simulink library "Garbage"  (R2023a)
settings: Solver=ode45  StartTime=0.0  StopTime=10.0  RelTol=1e-3  EnableMultiTasking=on
undefined symbols (not defined in the file or in its folder): c, ending_column_index, ending_row_index, framebuffer_16x1, framebuffer_2x2, framebuffer_4x4, framebuffer_8x8, HEIGHT, hot, POS_X, POS_Y, starting_column_index, starting_row_index, WIDTH
libraries: Memory_Blocks_Lib x459, simulink x235, simulink_extras x132, dspmtrx3 x116, Bit_Multiplexers_Lib x67, Bit_Demultiplexers_Lib x49, Byte_Multiplexers_Lib x40, Miscellaneous x38, Computer_Library x17, Decoders_Lib x14, commutil2 x12, Arithmetic_Operations_Lib x8
(part 12 of 26)

== system Garbage/NOT_USED_Counter_4bit_Hours ==
  note: J3 = ~(20+).~Rst.Q0.~Q3.Q2.Q1
      | K3 = (20+) + Rst + Q0 + ~Q3 + Q2 + Q1
      |
      | J2 = ~(20+).~Rst.Q0.~Q3.~Q2.Q1
      | K2 = ~(20+).~Rst.Q0.~Q3.Q2.Q1 + Rst + (20+) + Q3
      |
      | J1 = ~Rst.Q0.~Q3.(~(20+) + (20+).~Q2)
      | K1 = ((20+) + Rst + Q0 + Q3).(~(20+) + Rst + Q0 + Q3 + Q2)
      |
      | J0 = ~(20+).~Rst.~Q0.(~Q3 + Q3.~Q2.~Q1) + (20+).~Rst.~Q3.~Q2
      | K0 = 1
  note: Present state
      |
      | Next state
      |
      | Hours < 20
      | Hours >= 20
      | Hours < 20
      | Hours >= 20
      |
      | Rst = 0
      | Rst = 1
      | Rst = 0
      | Rst = 1
      | Rst = 0
      | Rst = 1
      | Rst = 0
      | Rst = 1
      |
      | Q3
      | Q2
      | Q1
      | Q0
      | J3
      | K3
      | J2
      | K2
      | J1
      | K1
      | J0
      | K0
      | J3
      | K3
      | J2
      | K2
      | J1
      | K1
      | J0
      | K0
      | J3
      | K3
      | J2
      | K2
      | J1
      | K1
      | J0
      | K0
      | J3
      | K3
      | J2
      | K2
      | J1
      | K1
      | J0
      | K0
      | Q3
      | Q2
      | Q1
      | Q0
      | Q3
      | Q2
      | Q1
      | Q0
      | Q3
      | Q2
      | Q1
      | Q0
      | Q3
      | Q2
      | Q1
      | Q0
      |
      | 0
      | 0
      | 0
      | 0
      | 0
      | X
      | 0
      | X
      | 0
      | X
      | 1
      | X
      | 0
      | X
      | 0
      | X
      | 0
      | X
      | 0
      | X
      | 0
      | X
      | 0
      | X
      | 0
      | X
      | 1
      | X
      | 0
      | X
      | 0
      | X
      | 0
      | X
      | 0
      | X
      | 0
      | 0
      | 0
      | 1
      | 0
      | 0
      | 0
      | 0
      | 0
      | 0
      | 0
      | 1
      | 0
      | 0
      | 0
      | 0
      |
      | 0
      | 0
      | 0
      | 1
      | 0
      | X
      | 0
      | X
      | 1
      | X
      | X
      | 1
      | 0
      | X
      | 0
      | X
      | 0
      | X
      | X
      | 1
      | 0
      | X
      | 0
      | X
      | 1
      | X
      | X
      | 1
      | 0
      | X
      | 0
      | X
      | 0
      | X
      | X
      | 1
      | 0
      | 0
      | 1
      | 0
      | 0
      | 0
      | 0
      | 0
      | 0
      | 0
      | 1
      | 0
      | 0
      | 0
      | 0
      | 0
      |
      | 0
      | 0
      | 1
      | 0
      | 0
      | X
      | 0
      | X
      | X
      | 0
      | 1
      | X
      | 0
      | X
      | 0
      | X
      | X
      | 1
      | 0
      | X
      | 0
      | X
      | 0
      | X
      | X
      | 0
      | 1
      | X
      | 0
      | X
      | 0
      | X
      | X
      | 1
      | 0
      | X
      | 0
      | 0
      | 1
      | 1
      | 0
      | 0
      | 0
      | 0
      | 0
      | 0
      | 1
      | 1
      | 0
      | 0
      | 0
      | 0
      |
      | 0
      | 0
      | 1
      | 1
      | 0
      | X
      | 1
      | X
      | X
      | 1
      | X
      | 1
      | 0
      | X
      | 0
      | X
      | X
      | 1
      | X
      | 1
      | 0
      | X
      | 0
      | X
      | X
      | 1
      | X
      | 1
      | 0
      | X
      | 0
      | X
      | X
      | 1
      | X
      | 1
      | 0
      | 1
      | 0
      | 0
      | 0
      | 0
      | 0
      | 0
      | 0
      | 0
      | 0
      | 0
      | 0
      | 0
      | 0
      | 0
      |
      | 0
      | 1
      | 0
      | 0
      | 0
      | X
      | X
      | 0
      | 0
      | X
      | 1
      | X
      | 0
      | X
      | X
      | 1
      | 0
      | X
      | 0
      | X
      | 0
      | X
      | X
      | 1
      | 0
      | X
      | 0
      | X
      | 0
      | X
      | X
      | 1
      | 0
      | X
      | 0
      | X
      | 0
      | 1
      | 0
      | 1
      | 0
      | 0
      | 0
      | 0
      | 0
      | 0
      | 0
      | 0
      | 0
      | 0
      | 0
      | 0
      |
      | 0
      | 1
      | 0
      | 1
      | 0
      | X
      | X
      | 0
      | 1
      | X
      | X
      | 1
      | 0
      | X
      | X
      | 1
      | 0
      | X
      | X
      | 1
      | 0
      | X
      | X
      | 1
      | 0
      | X
      | X
      | 1
      | 0
      | X
      | X
      | 1
      | 0
      | X
      | X
      | 1
      | 0
      | 1
      | 1
      | 0
      | 0
      | 0
      | 0
      | 0
      | 0
      | 0
      | 0
      | 0
      | 0
      | 0
      | 0
      | 0
      |
      | 0
      | 1
      | 1
      | 0
      | 0
      | X
      | X
      | 0
      | X
      | 0
      | 1
      | X
      | 0
      | X
      | X
      | 1
      | X
      | 1
      | 0
      | X
      | 0
      | X
      | X
      | 1
      | X
      | 1
      | 0
      | X
      | 0
      | X
      | X
      | 1
      | X
      | 1
      | 0
      | X
      | 0
      | 1
      | 1
      | 1
      | 0
      | 0
      | 0
      | 0
      | 0
      | 0
      | 0
      | 0
      | 0
      | 0
      | 0
      | 0
      |
      | 0
      | 1
      | 1
      | 1
      | 1
      | X
      | X
      | 1
      | X
      | 1
      | X
      | 1
      | 0
      | X
      | X
      | 1
      | X
      | 1
      | X
      | 1
      | 0
      | X
      | X
      | 1
      | X
      | 1
      | X
      | 1
      | 0
      | X
      | X
      | 1
      | X
      | 1
      | X
      | 1
      | 1
      | 0
      | 0
      | 0
      | 0
      | 0
      | 0
      | 0
      | 0
      | 0
      | 0
      | 0
      | 0
      | 0
      | 0
      | 0
      |
      | 1
      | 0
      | 0
      | 0
      | X
      | 0
      | 0
      | X
      | 0
      | X
      | 1
      | X
      | X
      | 1
      | 0
      | X
      | 0
      | X
      | 0
      | X
      | X
      | 1
      | 0
      | X
      | 0
      | X
      | 0
      | X
      | X
      | 1
      | 0
      | X
      | 0
      | X
      | 0
      | X
      | 1
      | 0
      | 0
      | 1
      | 0
      | 0
      | 0
      | 0
      | 0
      | 0
      | 0
      | 0
      | 0
      | 0
      | 0
      | 0
      |
      | 1
      | 0
      | 0
      | 1
      | X
      | 1
      | 0
      | X
      | 0
      | X
      | X
      | 1
      | X
      | 1
      | 0
      | X
      | 0
      | X
      | X
      | 1
      | X
      | 1
      | 0
      | X
      | 0
      | X
      | X
      | 1
      | X
      | 1
      | 0
      | X
      | 0
      | X
      | X
      | 1
      | 0
      | 0
      | 0
      | 0
      | 0
      | 0
      | 0
      | 0
      | 0
      | 0
      | 0
      | 0
      | 0
      | 0
      | 0
      | 0
      |
      | 1
      | 0
      | 1
      | 0
      | X
      | 1
      | 0
      | X
      | X
      | 1
      | 0
      | X
      | X
      | 1
      | 0
      | X
      | X
      | 1
      | 0
      | X
      | X
      | 1
      | 0
      | X
      | X
      | 1
      | 0
      | X
      | X
      | 1
      | 0
      | X
      | X
      | 1
      | 0
      | X
      | 0
      | 0
      | 0
      | 0
      | 0
      | 0
      | 0
      | 0
      | 0
      | 0
      | 0
      | 0
      | 0
      | 0
      | 0
      | 0
      |
      | 1
      | 0
      | 1
      | 1
      | X
      | 1
      | 0
      | X
      | X
      | 1
      | X
      | 1
      | X
      | 1
      | 0
      | X
      | X
      | 1
      | X
      | 1
      | X
      | 1
      | 0
      | X
      | X
      | 1
      | X
      | 1
      | X
      | 1
      | 0
      | X
      | X
      | 1
      | X
      | 1
      | 0
      | 0
      | 0
      | 0
      | 0
      | 0
      | 0
      | 0
      | 0
      | 0
      | 0
      | 0
      | 0
      | 0
      | 0
      | 0
      |
      | 1
      | 1
      | 0
      | 0
      | X
      | 1
      | X
      | 1
      | 0
      | X
      | 0
      | X
      | X
      | 1
      | X
      | 1
      | 0
      | X
      | 0
      | X
      | X
      | 1
      | X
      | 1
      | 0
      | X
      | 0
      | X
      | X
      | 1
      | X
      | 1
      | 0
      | X
      | 0
      | X
      | 0
      | 0
      | 0
      | 0
      | 0
      | 0
      | 0
      | 0
      | 0
      | 0
      | 0
      | 0
      | 0
      | 0
      | 0
      | 0
      |
      | 1
      | 1
      | 0
      | 1
      | X
      | 1
      | X
      | 1
      | 0
      | X
      | X
      | 1
      | X
      | 1
      | X
      | 1
      | 0
      | X
      | X
      | 1
      | X
      | 1
      | X
      | 1
      | 0
      | X
      | X
      | 1
      | X
      | 1
      | X
      | 1
      | 0
      | X
      | X
      | 1
      | 0
      | 0
      | 0
      | 0
      | 0
      | 0
      | 0
      | 0
      | 0
      | 0
      | 0
      | 0
      | 0
      | 0
      | 0
      | 0
      |
      | 1
      | 1
      | 1
      | 0
      | X
      | 1
      | X
      | 1
      | X
      | 1
      | 0
      | X
      | X
      | 1
      | X
      | 1
      | X
      | 1
      | 0
      | X
      | X
      | 1
      | X
      | 1
      | X
      | 1
      | 0
      | X
      | X
      | 1
      | X
      | 1
      | X
      | 1
      | 0
      | X
      | 0
      | 0
      | 0
      | 0
      | 0
      | 0
      | 0
      | 0
      | 0
      | 0
      | 0
      | 0
      | 0
      | 0
      | 0
      | 0
      |
      | 1
      | 1
      | 1
      | 1
      | X
      | 1
      | X
      | 1
      | X
      | 1
      | X
      | 1
      | X
      | 1
      | X
      | 1
      | X
      | 1
      | X
      | 1
      | X
      | 1
      | X
      | 1
      | X
      | 1
      | X
      | 1
      | X
      | 1
      | X
      | 1
      | X
      | 1
      | X
      | 1
      | 0
      | 0
      | 0
      | 0
      | 0
      | 0
      | 0
      | 0
      | 0
      | 0
      | 0
      | 0
      | 0
      | 0
      | 0
      | 0
  note: 4-bit counter with reset
      | If (Hours < 20) then
      | (0 -> 1 -> 2 -> 3 -> 4 -> 5 -> 6 -> 7 -> 8 -> 9 -> 0)
      |
      | If (Hours >= 20) then
      | (0 -> 1 -> 2 -> 3 -> 0)
  note: K3
      | Q3/Q2/Q1
      |
      | 000
      | 001
      | 011
      | 010
      | 110
      | 111
      | 101
      | 100
      |
      | 20+/Rst/Q0
      | 000
      | x
      | x
      | x
      | x
      | 1
      | 1
      | 1
      | 0
      |
      | 001
      | x
      | x
      | x
      | x
      | 1
      | 1
      | 1
      | 1
      |
      | 011
      | x
      | x
      | x
      | x
      | 1
      | 1
      | 1
      | 1
      |
      | 010
      | x
      | x
      | x
      | x
      | 1
      | 1
      | 1
      | 1
      |
      | 110
      | x
      | x
      | x
      | x
      | 1
      | 1
      | 1
      | 1
      |
      | 111
      | x
      | x
      | x
      | x
      | 1
      | 1
      | 1
      | 1
      |
      | 101
      | x
      | x
      | x
      | x
      | 1
      | 1
      | 1
      | 1
      |
      | 100
      | x
      | x
      | x
      | x
      | 1
      | 1
      | 1
      | 1
      |
      | K3 = (20+) + Rst + Q0 + ~Q3 + Q2 + Q1
  note: J2
      | Q3/Q2/Q1
      |
      | 000
      | 001
      | 011
      | 010
      | 110
      | 111
      | 101
      | 100
      |
      | 20+/Rst/Q0
      | 000
      | 0
      | 0
      | x
      | x
      | x
      | x
      | 0
      | 0
      |
      | 001
      | 0
      | 1
      | x
      | x
      | x
      | x
      | 0
      | 0
      |
      | 011
      | 0
      | 0
      | x
      | x
      | x
      | x
      | 0
      | 0
      |
      | 010
      | 0
      | 0
      | x
      | x
      | x
      | x
      | 0
      | 0
      |
      | 110
      | 0
      | 0
      | x
      | x
      | x
      | x
      | 0
      | 0
      |
      | 111
      | 0
      | 0
      | x
      | x
      | x
      | x
      | 0
      | 0
      |
      | 101
      | 0
      | 0
      | x
      | x
      | x
      | x
      | 0
      | 0
      |
      | 100
      | 0
      | 0
      | x
      | x
      | x
      | x
      | 0
      | 0
      |
      | J2 = ~(20+).~Rst.Q0.~Q3.~Q2.Q1
  note: K2
      | Q3/Q2/Q1
      |
      | 000
      | 001
      | 011
      | 010
      | 110
      | 111
      | 101
      | 100
      |
      | 20+/Rst/Q0
      | 000
      | x
      | x
      | 0
      | 0
      | 1
      | 1
      | x
      | x
      |
      | 001
      | x
      | x
      | 1
      | 0
      | 1
      | 1
      | x
      | x
      |
      | 011
      | x
      | x
      | 1
      | 1
      | 1
      | 1
      | x
      | x
      |
      | 010
      | x
      | x
      | 1
      | 1
      | 1
      | 1
      | x
      | x
      |
      | 110
      | x
      | x
      | 1
      | 1
      | 1
      | 1
      | x
      | x
      |
      | 111
      | x
      | x
      | 1
      | 1
      | 1
      | 1
      | x
      | x
      |
      | 101
      | x
      | x
      | 1
      | 1
      | 1
      | 1
      | x
      | x
      |
      | 100
      | x
      | x
      | 1
      | 1
      | 1
      | 1
      | x
      | x
      |
      | K2 = ~(20+).~Rst.Q0.~Q3.Q2.Q1 + Rst + (20+) + Q3
  note: J1
      | Q3/Q2/Q1
      |
      | 000
      | 001
      | 011
      | 010
      | 110
      | 111
      | 101
      | 100
      |
      | 20+/Rst/Q0
      | 000
      | 0
      | x
      | x
      | 0
      | 0
      | x
      | x
      | 0
      |
      | 001
      | 1
      | x
      | x
      | 1
      | 0
      | x
      | x
      | 0
      |
      | 011
      | 0
      | x
      | x
      | 0
      | 0
      | x
      | x
      | 0
      |
      | 010
      | 0
      | x
      | x
      | 0
      | 0
      | x
      | x
      | 0
      |
      | 110
      | 0
      | x
      | x
      | 0
      | 0
      | x
      | x
      | 0
      |
      | 111
      | 0
      | x
      | x
      | 0
      | 0
      | x
      | x
      | 0
      |
      | 101
      | 1
      | x
      | x
      | 0
      | 0
      | x
      | x
      | 0
      |
      | 100
      | 0
      | x
      | x
      | 0
      | 0
      | x
      | x
      | 0
      |
      | J1 = ~(20+).~Rst.Q0.~Q3 + (20+).~Rst.Q0.~Q3.~Q2 =
      | = ~Rst.Q0.~Q3.(~(20+) + (20+).~Q2)
  note: K1
      | Q3/Q2/Q1
      |
      | 000
      | 001
      | 011
      | 010
      | 110
      | 111
      | 101
      | 100
      |
      | 20+/Rst/Q0
      | 000
      | x
      | 0
      | 0
      | x
      | x
      | 1
      | 1
      | x
      |
      | 001
      | x
      | 1
      | 1
      | x
      | x
      | 1
      | 1
      | x
      |
      | 011
      | x
      | 1
      | 1
      | x
      | x
      | 1
      | 1
      | x
      |
      | 010
      | x
      | 1
      | 1
      | x
      | x
      | 1
      | 1
      | x
      |
      | 110
      | x
      | 1
      | 1
      | x
      | x
      | 1
      | 1
      | x
      |
      | 111
      | x
      | 1
      | 1
      | x
      | x
      | 1
      | 1
      | x
      |
      | 101
      | x
      | 1
      | 1
      | x
      | x
      | 1
      | 1
      | x
      |
      | 100
      | x
      | 0
      | 1
      | x
      | x
      | 1
      | 1
      | x
      |
      | K1 = ((20+) + Rst + Q0 + Q3).(~(20+) + Rst + Q0 + Q3 + Q2)
  note: J0
      | Q3/Q2/Q1
      |
      | 000
      | 001
      | 011
      | 010
      | 110
      | 111
      | 101
      | 100
      |
      | 20+/Rst/Q0
      | 000
      | 1
      | 1
      | 1
      | 1
      | 0
      | 0
      | 0
      | 1
      |
      | 001
      | x
      | x
      | x
      | x
      | x
      | x
      | x
      | x
      |
      | 011
      | x
      | x
      | x
      | x
      | x
      | x
      | x
      | x
      |
      | 010
      | 0
      | 0
      | 0
      | 0
      | 0
      | 0
      | 0
      | 0
      |
      | 110
      | 0
      | 0
      | 0
      | 0
      | 0
      | 0
      | 0
      | 0
      |
      | 111
      | x
      | x
      | x
      | x
      | x
      | x
      | x
      | x
      |
      | 101
      | x
      | x
      | x
      | x
      | x
      | x
      | x
      | x
      |
      | 100
      | 1
      | 1
      | 0
      | 0
      | 0
      | 0
      | 0
      | 0
      |
      | J0 = ~(20+).~Rst.~Q0.(~Q3 + Q3.~Q2.~Q1) + (20+).~Rst.~Q3.~Q2
      | K0 = 1
  note: J3
      | Q3/Q2/Q1
      |
      | 000
      | 001
      | 011
      | 010
      | 110
      | 111
      | 101
      | 100
      |
      | 20+/Rst/Q0
      | 000
      | 0
      | 0
      | 0
      | 0
      | x
      | x
      | x
      | x
      |
      | 001
      | 0
      | 0
      | 1
      | 0
      | x
      | x
      | x
      | x
      |
      | 011
      | 0
      | 0
      | 0
      | 0
      | x
      | x
      | x
      | x
      |
      | 010
      | 0
      | 0
      | 0
      | 0
      | x
      | x
      | x
      | x
      |
      | 110
      | 0
      | 0
      | 0
      | 0
      | x
      | x
      | x
      | x
      |
      | 111
      | 0
      | 0
      | 0
      | 0
      | x
      | x
      | x
      | x
      |
      | 101
      | 0
      | 0
      | 0
      | 0
      | x
      | x
      | x
      | x
      |
      | 100
      | 0
      | 0
      | 0
      | 0
      | x
      | x
      | x
      | x
      |
      | J3 = ~(20+).~Rst.Q0.~Q3.Q2.Q1
  Reset : Inport
  20+ : Inport  Port=2
  CLK : Inport  Port=3
  Bit_{0..3} (4 alike) : simulink_extras/Flip Flops/J-K Flip-Flop  initial_condition=0
  Constant3 : Constant  OutDataTypeStr=boolean
  Logical Operator : Logic  Operator=NOT  IconShape=distinctive  AllPortsSameDT=off  OutDataTypeStr=boolean
  Logical Operator1 : Logic  Inputs=4  IconShape=distinctive  AllPortsSameDT=off  OutDataTypeStr=boolean
  Logical Operator10 : Logic  Inputs=6  IconShape=distinctive  AllPortsSameDT=off  OutDataTypeStr=boolean
  Logical Operator11 : Logic  Operator=OR  IconShape=distinctive  AllPortsSameDT=off  OutDataTypeStr=boolean
  Logical Operator12 : Logic  Operator=NOT  IconShape=distinctive  AllPortsSameDT=off  OutDataTypeStr=boolean
  Logical Operator13 : Logic  Inputs=4  IconShape=distinctive  AllPortsSameDT=off  OutDataTypeStr=boolean
  Logical Operator14 : Logic  Operator=OR  IconShape=distinctive  AllPortsSameDT=off  OutDataTypeStr=boolean
  Logical Operator15 : Logic  IconShape=distinctive  AllPortsSameDT=off  OutDataTypeStr=boolean
  Logical Operator16 : Logic  IconShape=distinctive  AllPortsSameDT=off  OutDataTypeStr=boolean
  Logical Operator17 : Logic  Operator=OR  Inputs=5  IconShape=distinctive  AllPortsSameDT=off  OutDataTypeStr=boolean
  Logical Operator2 : Logic  Inputs=4  IconShape=distinctive  AllPortsSameDT=off  OutDataTypeStr=boolean
  Logical Operator3 : Logic  Operator=OR  Inputs=6  IconShape=distinctive  AllPortsSameDT=off  OutDataTypeStr=boolean
  Logical Operator4 : Logic  Operator=OR  IconShape=distinctive  AllPortsSameDT=off  OutDataTypeStr=boolean
  Logical Operator5 : Logic  Inputs=6  IconShape=distinctive  AllPortsSameDT=off  OutDataTypeStr=boolean
  Logical Operator6 : Logic  Inputs=6  IconShape=distinctive  AllPortsSameDT=off  OutDataTypeStr=boolean
  Logical Operator7 : Logic  Operator=OR  Inputs=4  IconShape=distinctive  AllPortsSameDT=off  OutDataTypeStr=boolean
  Logical Operator8 : Logic  Operator=OR  Inputs=4  IconShape=distinctive  AllPortsSameDT=off  OutDataTypeStr=boolean
  Logical Operator9 : Logic  Inputs=3  IconShape=distinctive  AllPortsSameDT=off  OutDataTypeStr=boolean
  Bit3 : Outport
  Bit2 : Outport  Port=2
  Bit1 : Outport  Port=3
  Bit0 : Outport  Port=4
  wires:
  CLK/1 -> Bit_3/2, Bit_2/2, Bit_1/2, Bit_0/2
  Constant3/1 -> Bit_0/3
  Logical Operator10/1 -> Bit_3/1
  Logical Operator3/1 -> Bit_3/3
  Bit_3/2 -> Logical Operator5/6, Logical Operator1/3, Logical Operator4/2, Logical Operator13/4, Logical Operator3/5, Logical Operator10/5, Logical Operator6/5
  Bit_2/1 -> Logical Operator6/2, Logical Operator3/1, Logical Operator10/4, Bit2/1, Logical Operator17/4
  Bit_0/1 -> Logical Operator7/2, Logical Operator17/5, Logical Operator1/2, Logical Operator5/3, Logical Operator6/4, Logical Operator3/3, Logical Operator10/2, Bit0/1
  Logical Operator/1 -> Logical Operator10/1, Logical Operator5/5, Logical Operator6/1, Logical Operator1/1, Logical Operator2/1, Logical Operator13/2
  Logical Operator5/1 -> Bit_2/1
  Bit_2/2 -> Logical Operator5/1, Logical Operator15/2, Logical Operator9/3, Logical Operator13/3
  Logical Operator8/1 -> Bit_2/3
  Bit_3/1 -> Logical Operator8/3, Logical Operator7/4, Logical Operator17/1, Logical Operator9/1, Bit3/1
  Logical Operator6/1 -> Logical Operator8/4
  Bit_1/1 -> Logical Operator5/4, Logical Operator6/3, Logical Operator3/2, Logical Operator10/3, Bit1/1
  Logical Operator1/1 -> Bit_1/1
  Logical Operator7/1 -> Logical Operator16/1
  Reset/1 -> Logical Operator/1, Logical Operator3/4, Logical Operator8/2, Logical Operator17/2, Logical Operator7/3
  Logical Operator4/1 -> Logical Operator2/3
  Logical Operator2/1 -> Logical Operator11/2
  Bit_0/2 -> Logical Operator2/4
  Logical Operator9/1 -> Logical Operator4/1
  Bit_1/2 -> Logical Operator9/2
  Logical Operator11/1 -> Bit_0/1
  20+/1 -> Logical Operator12/1, Logical Operator8/1, Logical Operator15/1, Logical Operator7/1, Logical Operator13/1, Logical Operator3/6
  Logical Operator12/1 -> Logical Operator17/3, Logical Operator14/1, Logical Operator2/2, Logical Operator5/2, Logical Operator6/6, Logical Operator10/6
  Logical Operator13/1 -> Logical Operator11/1
  Logical Operator14/1 -> Logical Operator1/4
  Logical Operator15/1 -> Logical Operator14/2
  Logical Operator16/1 -> Bit_1/3
  Logical Operator17/1 -> Logical Operator16/2

== system Garbage/NOT_USED_Decoder_6_to_64 ==
  Addr(1B) : Inport
  Lo`gical Operator1 : Logic  Operator=NOT  IconShape=distinctive  AllPortsSameDT=off  OutDataTypeStr=boolean
  Lo`gical Operator2 : Logic  Operator=NOT  IconShape=distinctive  AllPortsSameDT=off  OutDataTypeStr=boolean
  Lo`gical Operator4 : Logic  Operator=NOT  IconShape=distinctive  AllPortsSameDT=off  OutDataTypeStr=boolean
  Logical Operator{,1..4,6..21,24..66} (64 alike) : Logic  Inputs=6  IconShape=distinctive  AllPortsSameDT=off  OutDataTypeStr=boolean
  Logical Operator22 : Logic  Operator=NOT  IconShape=distinctive  AllPortsSameDT=off  OutDataTypeStr=boolean
  Logical Operator23 : Logic  Operator=NOT  IconShape=distinctive  AllPortsSameDT=off  OutDataTypeStr=boolean
  Logical Operator5 : Logic  Operator=NOT  IconShape=distinctive  AllPortsSameDT=off  OutDataTypeStr=boolean
  Terminator : Terminator
  Terminator1 : Terminator
  xyz1 : BusSelector  OutputSignals=signal1,signal2,signal3,signal4,signal5,signal6,signal7,signal8
  Select_63(1b) : Outport
  Select_62(1b) : Outport  Port=2
  Select_61(1b) : Outport  Port=3
  Select_60(1b) : Outport  Port=4
  Select_59(1b) : Outport  Port=5
  Select_58(1b) : Outport  Port=6
  Select_57(1b) : Outport  Port=7
  Select_56(1b) : Outport  Port=8
  Select_55(1b) : Outport  Port=9
  Select_54(1b) : Outport  Port=10
  Select_53(1b) : Outport  Port=11
  Select_52(1b) : Outport  Port=12
  Select_51(1b) : Outport  Port=13
  Select_50(1b) : Outport  Port=14
  Select_49(1b) : Outport  Port=15
  Select_48(1b) : Outport  Port=16
  Select_47(1b) : Outport  Port=17
  Select_46(1b) : Outport  Port=18
  Select_45(1b) : Outport  Port=19
  Select_44(1b) : Outport  Port=20
  Select_43(1b) : Outport  Port=21
  Select_42(1b) : Outport  Port=22
  Select_41(1b) : Outport  Port=23
  Select_40(1b) : Outport  Port=24
  Select_39(1b) : Outport  Port=25
  Select_38(1b) : Outport  Port=26
  Select_37(1b) : Outport  Port=27
  Select_36(1b) : Outport  Port=28
  Select_35(1b) : Outport  Port=29
  Select_34(1b) : Outport  Port=30
  Select_33(1b) : Outport  Port=31
  Select_32(1b) : Outport  Port=32
  Select_31(1b) : Outport  Port=33
  Select_30(1b) : Outport  Port=34
  Select_29(1b) : Outport  Port=35
  Select_28(1b) : Outport  Port=36
  Select_27(1b) : Outport  Port=37
  Select_26(1b) : Outport  Port=38
  Select_25(1b) : Outport  Port=39
  Select_24(1b) : Outport  Port=40
  Select_23(1b) : Outport  Port=41
  Select_22(1b) : Outport  Port=42
  Select_21(1b) : Outport  Port=43
  Select_20(1b) : Outport  Port=44
  Select_19(1b) : Outport  Port=45
  Select_18(1b) : Outport  Port=46
  Select_17(1b) : Outport  Port=47
  Select_16(1b) : Outport  Port=48
  Select_15(1b) : Outport  Port=49
  Select_14(1b) : Outport  Port=50
  Select_13(1b) : Outport  Port=51
  Select_12(1b) : Outport  Port=52
  Select_11(1b) : Outport  Port=53
  Select_10(1b) : Outport  Port=54
  Select_09(1b) : Outport  Port=55
  Select_08(1b) : Outport  Port=56
  Select_07(1b) : Outport  Port=57
  Select_06(1b) : Outport  Port=58
  Select_05(1b) : Outport  Port=59
  Select_04(1b) : Outport  Port=60
  Select_03(1b) : Outport  Port=61
  Select_02(1b) : Outport  Port=62
  Select_01(1b) : Outport  Port=63
  Select_00(1b) : Outport  Port=64
  wires:
  Logical Operator16/1 -> Select_48(1b)/1
  Logical Operator15/1 -> Select_49(1b)/1
  Logical Operator14/1 -> Select_50(1b)/1
  Logical Operator13/1 -> Select_51(1b)/1
  Logical Operator12/1 -> Select_52(1b)/1
  Logical Operator11/1 -> Select_53(1b)/1
  Logical Operator10/1 -> Select_54(1b)/1
  Logical Operator9/1 -> Select_55(1b)/1
  Logical Operator8/1 -> Select_56(1b)/1
  Logical Operator7/1 -> Select_57(1b)/1
  Logical Operator6/1 -> Select_58(1b)/1
  Logical Operator4/1 -> Select_59(1b)/1
  Logical Operator1/1 -> Select_62(1b)/1
  Logical Operator2/1 -> Select_61(1b)/1
  Logical Operator3/1 -> Select_60(1b)/1
  Logical Operator/1 -> Select_63(1b)/1
  Logical Operator27/1 -> Select_32(1b)/1
  Logical Operator26/1 -> Select_33(1b)/1
  Logical Operator25/1 -> Select_34(1b)/1
  Logical Operator24/1 -> Select_35(1b)/1
  Logical Operator21/1 -> Select_36(1b)/1
  Logical Operator20/1 -> Select_37(1b)/1
  Logical Operator19/1 -> Select_38(1b)/1
  Logical Operator34/1 -> Select_39(1b)/1
  Logical Operator33/1 -> Select_40(1b)/1
  Logical Operator32/1 -> Select_41(1b)/1
  Logical Operator31/1 -> Select_42(1b)/1
  Logical Operator30/1 -> Select_43(1b)/1
  Logical Operator18/1 -> Select_46(1b)/1
  Logical Operator28/1 -> Select_45(1b)/1
  Logical Operator29/1 -> Select_44(1b)/1
  Logical Operator17/1 -> Select_47(1b)/1
  xyz1/3 -> Logical Operator/1, Logical Operator1/1, Logical Operator2/1, Logical Operator3/1, Logical Operator4/1, Logical Operator6/1, Logical Operator7/1, Logical Operator8/1, Logical Operator9/1, Logical Operator10/1, Logical Operator11/1, Logical Operator12/1, Logical Operator13/1, Logical Operator14/1, Logical Operator15/1, Logical Operator16/1, Logical Operator17/1, Logical Operator18/1, Logical Operator28/1, Logical Operator29/1, Logical Operator30/1, Logical Operator31/1, Logical Operator32/1, Logical Operator33/1, Logical Operator34/1, Logical Operator19/1, Logical Operator20/1, Logical Operator21/1, Logical Operator24/1, Logical Operator25/1, Logical Operator27/1, Logical Operator26/1, Lo`gical Operator2/1  "<signal3>"
  Logical Operator43/1 -> Select_16(1b)/1
  Logical Operator42/1 -> Select_17(1b)/1
  Logical Operator41/1 -> Select_18(1b)/1
  Logical Operator40/1 -> Select_19(1b)/1
  Logical Operator39/1 -> Select_20(1b)/1
  Logical Operator38/1 -> Select_21(1b)/1
  Logical Operator37/1 -> Select_22(1b)/1
  Logical Operator50/1 -> Select_23(1b)/1
  Logical Operator49/1 -> Select_24(1b)/1
  Logical Operator48/1 -> Select_25(1b)/1
  Logical Operator47/1 -> Select_26(1b)/1
  Logical Operator46/1 -> Select_27(1b)/1
  Logical Operator36/1 -> Select_30(1b)/1
  Logical Operator44/1 -> Select_29(1b)/1
  Logical Operator45/1 -> Select_28(1b)/1
  Logical Operator35/1 -> Select_31(1b)/1
  Logical Operator22/1 -> Logical Operator2/5, Logical Operator3/5, Logical Operator7/5, Logical Operator8/5, Logical Operator11/5, Logical Operator12/5, Logical Operator15/5, Logical Operator16/5, Logical Operator28/5, Logical Operator29/5, Logical Operator32/5, Logical Operator33/5, Logical Operator20/5, Logical Operator21/5, Logical Operator26/5, Logical Operator27/5, Logical Operator44/5, Logical Operator45/5, Logical Operator48/5, Logical Operator49/5, Logical Operator38/5, Logical Operator39/5, Logical Operator42/5, Logical Operator43/5, Logical Operator60/5, Logical Operator61/5, Logical Operator64/5, Logical Operator65/5, Logical Operator54/5, Logical Operator55/5, Logical Operator59/5, Logical Operator58/5
  xyz1/8 -> Logical Operator/6, Logical Operator2/6, Logical Operator4/6, Logical Operator7/6, Logical Operator9/6, Logical Operator11/6, Logical Operator13/6, Logical Operator15/6, Logical Operator17/6, Logical Operator28/6, Logical Operator30/6, Logical Operator32/6, Logical Operator34/6, Logical Operator20/6, Logical Operator24/6, Logical Operator26/6, Logical Operator35/6, Logical Operator44/6, Logical Operator46/6, Logical Operator48/6, Logical Operator50/6, Logical Operator38/6, Logical Operator40/6, Logical Operator42/6, Logical Operator51/6, Logical Operator60/6, Logical Operator62/6, Logical Operator64/6, Logical Operator66/6, Logical Operator54/6, Logical Operator58/6, Logical Operator56/6, Logical Operator23/1  "<signal8>"
  Logical Operator23/1 -> Logical Operator1/6, Logical Operator3/6, Logical Operator6/6, Logical Operator8/6, Logical Operator10/6, Logical Operator12/6, Logical Operator14/6, Logical Operator16/6, Logical Operator18/6, Logical Operator29/6, Logical Operator31/6, Logical Operator33/6, Logical Operator19/6, Logical Operator21/6, Logical Operator25/6, Logical Operator27/6, Logical Operator36/6, Logical Operator45/6, Logical Operator47/6, Logical Operator49/6, Logical Operator37/6, Logical Operator39/6, Logical Operator41/6, Logical Operator43/6, Logical Operator52/6, Logical Operator61/6, Logical Operator63/6, Logical Operator65/6, Logical Operator53/6, Logical Operator55/6, Logical Operator59/6, Logical Operator57/6
  Logical Operator5/1 -> Logical Operator4/4, Logical Operator6/4, Logical Operator7/4, Logical Operator8/4, Logical Operator13/4, Logical Operator14/4, Logical Operator15/4, Logical Operator16/4, Logical Operator30/4, Logical Operator31/4, Logical Operator32/4, Logical Operator33/4, Logical Operator24/4, Logical Operator25/4, Logical Operator26/4, Logical Operator27/4, Logical Operator46/4, Logical Operator47/4, Logical Operator48/4, Logical Operator49/4, Logical Operator40/4, Logical Operator41/4, Logical Operator42/4, Logical Operator43/4, Logical Operator62/4, Logical Operator63/4, Logical Operator64/4, Logical Operator65/4, Logical Operator56/4, Logical Operator57/4, Logical Operator59/4, Logical Operator58/4
  xyz1/5 -> Lo`gical Operator4/1, Logical Operator/3, Logical Operator1/3, Logical Operator2/3, Logical Operator3/3, Logical Operator4/3, Logical Operator6/3, Logical Operator7/3, Logical Operator8/3, Logical Operator17/3, Logical Operator18/3, Logical Operator28/3, Logical Operator29/3, Logical Operator30/3, Logical Operator31/3, Logical Operator32/3, Logical Operator33/3, Logical Operator35/3, Logical Operator36/3, Logical Operator44/3, Logical Operator45/3, Logical Operator46/3, Logical Operator47/3, Logical Operator48/3, Logical Operator49/3, Logical Operator51/3, Logical Operator52/3, Logical Operator60/3, Logical Operator61/3, Logical Operator62/3, Logical Operator63/3, Logical Operator64/3, Logical Operator65/3  "<signal5>"
  xyz1/7 -> Logical Operator22/1, Logical Operator/5, Logical Operator1/5, Logical Operator4/5, Logical Operator6/5, Logical Operator9/5, Logical Operator10/5, Logical Operator13/5, Logical Operator14/5, Logical Operator17/5, Logical Operator18/5, Logical Operator30/5, Logical Operator31/5, Logical Operator34/5, Logical Operator19/5, Logical Operator24/5, Logical Operator25/5, Logical Operator35/5, Logical Operator36/5, Logical Operator46/5, Logical Operator47/5, Logical Operator50/5, Logical Operator37/5, Logical Operator40/5, Logical Operator41/5, Logical Operator51/5, Logical Operator52/5, Logical Operator62/5, Logical Operator63/5, Logical Operator66/5, Logical Operator53/5, Logical Operator57/5, Logical Operator56/5  "<signal7>"
  xyz1/6 -> Logical Operator5/1, Logical Operator/4, Logical Operator1/4, Logical Operator2/4, Logical Operator3/4, Logical Operator9/4, Logical Operator10/4, Logical Operator11/4, Logical Operator12/4, Logical Operator17/4, Logical Operator18/4, Logical Operator28/4, Logical Operator29/4, Logical Operator34/4, Logical Operator19/4, Logical Operator20/4, Logical Operator21/4, Logical Operator35/4, Logical Operator36/4, Logical Operator44/4, Logical Operator45/4, Logical Operator50/4, Logical Operator37/4, Logical Operator38/4, Logical Operator39/4, Logical Operator51/4, Logical Operator52/4, Logical Operator60/4, Logical Operator61/4, Logical Operator66/4, Logical Operator53/4, Logical Operator55/4, Logical Operator54/4  "<signal6>"
  Lo`gical Operator4/1 -> Logical Operator9/3, Logical Operator10/3, Logical Operator11/3, Logical Operator12/3, Logical Operator13/3, Logical Operator14/3, Logical Operator15/3, Logical Operator16/3, Logical Operator34/3, Logical Operator19/3, Logical Operator20/3, Logical Operator21/3, Logical Operator24/3, Logical Operator25/3, Logical Operator26/3, Logical Operator27/3, Logical Operator50/3, Logical Operator37/3, Logical Operator38/3, Logical Operator39/3, Logical Operator40/3, Logical Operator41/3, Logical Operator42/3, Logical Operator43/3, Logical Operator66/3, Logical Operator53/3, Logical Operator54/3, Logical Operator55/3, Logical Operator56/3, Logical Operator57/3, Logical Operator59/3, Logical Operator58/3
  Logical Operator59/1 -> Select_00(1b)/1
  Logical Operator58/1 -> Select_01(1b)/1
  Logical Operator57/1 -> Select_02(1b)/1
  Logical Operator56/1 -> Select_03(1b)/1
  Logical Operator55/1 -> Select_04(1b)/1
  Logical Operator54/1 -> Select_05(1b)/1
  Logical Operator53/1 -> Select_06(1b)/1
  Logical Operator66/1 -> Select_07(1b)/1
  Logical Operator65/1 -> Select_08(1b)/1
  Logical Operator64/1 -> Select_09(1b)/1
  Logical Operator63/1 -> Select_10(1b)/1
  Logical Operator62/1 -> Select_11(1b)/1
  Logical Operator52/1 -> Select_14(1b)/1
  Logical Operator60/1 -> Select_13(1b)/1
  Logical Operator61/1 -> Select_12(1b)/1
  Logical Operator51/1 -> Select_15(1b)/1
  Lo`gical Operator1/1 -> Logical Operator17/2, Logical Operator18/2, Logical Operator28/2, Logical Operator29/2, Logical Operator30/2, Logical Operator31/2, Logical Operator32/2, Logical Operator33/2, Logical Operator34/2, Logical Operator19/2, Logical Operator20/2, Logical Operator21/2, Logical Operator24/2, Logical Operator25/2, Logical Operator26/2, Logical Operator27/2, Logical Operator51/2, Logical Operator52/2, Logical Operator60/2, Logical Operator61/2, Logical Operator62/2, Logical Operator63/2, Logical Operator64/2, Logical Operator65/2, Logical Operator66/2, Logical Operator53/2, Logical Operator54/2, Logical Operator55/2, Logical Operator56/2, Logical Operator57/2, Logical Operator59/2, Logical Operator58/2
  xyz1/4 -> Logical Operator/2, Logical Operator1/2, Logical Operator2/2, Logical Operator3/2, Logical Operator4/2, Logical Operator6/2, Logical Operator7/2, Logical Operator8/2, Logical Operator9/2, Logical Operator10/2, Logical Operator11/2, Logical Operator12/2, Logical Operator13/2, Logical Operator14/2, Logical Operator15/2, Logical Operator16/2, Logical Operator35/2, Logical Operator36/2, Logical Operator44/2, Logical Operator45/2, Logical Operator46/2, Logical Operator47/2, Logical Operator48/2, Logical Operator49/2, Logical Operator50/2, Logical Operator37/2, Logical Operator38/2, Logical Operator39/2, Logical Operator40/2, Logical Operator41/2, Logical Operator43/2, Logical Operator42/2, Lo`gical Operator1/1  "<signal4>"
  Lo`gical Operator2/1 -> Logical Operator35/1, Logical Operator36/1, Logical Operator44/1, Logical Operator45/1, Logical Operator46/1, Logical Operator47/1, Logical Operator48/1, Logical Operator49/1, Logical Operator50/1, Logical Operator37/1, Logical Operator38/1, Logical Operator39/1, Logical Operator40/1, Logical Operator41/1, Logical Operator42/1, Logical Operator43/1, Logical Operator51/1, Logical Operator52/1, Logical Operator60/1, Logical Operator61/1, Logical Operator62/1, Logical Operator63/1, Logical Operator64/1, Logical Operator65/1, Logical Operator66/1, Logical Operator53/1, Logical Operator54/1, Logical Operator55/1, Logical Operator56/1, Logical Operator57/1, Logical Operator59/1, Logical Operator58/1
  Addr(1B)/1 -> xyz1/1
  xyz1/1 -> Terminator/1  "<signal1>"
  xyz1/2 -> Terminator1/1  "<signal2>"

== system Garbage/NOT_USED_Decoder_6_to_64_Enable ==
  Enable(1b) : Inport
  Addr(1B) : Inport  Port=2
  Lo`gical Operator1 : Logic  Operator=NOT  IconShape=distinctive  AllPortsSameDT=off  OutDataTypeStr=boolean
  Lo`gical Operator2 : Logic  Operator=NOT  IconShape=distinctive  AllPortsSameDT=off  OutDataTypeStr=boolean
  Lo`gical Operator4 : Logic  Operator=NOT  IconShape=distinctive  AllPortsSameDT=off  OutDataTypeStr=boolean
  Logical Operator{,1..4,6..21,24..49,52..64,66..69} (64 alike) : Logic  Inputs=7  IconShape=distinctive  AllPortsSameDT=off  OutDataTypeStr=boolean
  Logical Operator22 : Logic  Operator=NOT  IconShape=distinctive  AllPortsSameDT=off  OutDataTypeStr=boolean
  Logical Operator23 : Logic  Operator=NOT  IconShape=distinctive  AllPortsSameDT=off  OutDataTypeStr=boolean
  Logical Operator5 : Logic  Operator=NOT  IconShape=distinctive  AllPortsSameDT=off  OutDataTypeStr=boolean
  Terminator : Terminator
  Terminator1 : Terminator
  xyz1 : BusSelector  OutputSignals=signal1,signal2,signal3,signal4,signal5,signal6,signal7,signal8
  Select_63(1b) : Outport
  Select_62(1b) : Outport  Port=2
  Select_61(1b) : Outport  Port=3
  Select_60(1b) : Outport  Port=4
  Select_59(1b) : Outport  Port=5
  Select_58(1b) : Outport  Port=6
  Select_57(1b) : Outport  Port=7
  Select_56(1b) : Outport  Port=8
  Select_55(1b) : Outport  Port=9
  Select_54(1b) : Outport  Port=10
  Select_53(1b) : Outport  Port=11
  Select_52(1b) : Outport  Port=12
  Select_51(1b) : Outport  Port=13
  Select_50(1b) : Outport  Port=14
  Select_49(1b) : Outport  Port=15
  Select_48(1b) : Outport  Port=16
  Select_47(1b) : Outport  Port=17
  Select_46(1b) : Outport  Port=18
  Select_45(1b) : Outport  Port=19
  Select_44(1b) : Outport  Port=20
  Select_43(1b) : Outport  Port=21
  Select_42(1b) : Outport  Port=22
  Select_41(1b) : Outport  Port=23
  Select_40(1b) : Outport  Port=24
  Select_39(1b) : Outport  Port=25
  Select_38(1b) : Outport  Port=26
  Select_37(1b) : Outport  Port=27
  Select_36(1b) : Outport  Port=28
  Select_35(1b) : Outport  Port=29
  Select_34(1b) : Outport  Port=30
  Select_33(1b) : Outport  Port=31
  Select_32(1b) : Outport  Port=32
  Select_31(1b) : Outport  Port=33
  Select_30(1b) : Outport  Port=34
  Select_29(1b) : Outport  Port=35
  Select_28(1b) : Outport  Port=36
  Select_27(1b) : Outport  Port=37
  Select_26(1b) : Outport  Port=38
  Select_25(1b) : Outport  Port=39
  Select_24(1b) : Outport  Port=40
  Select_23(1b) : Outport  Port=41
  Select_22(1b) : Outport  Port=42
  Select_21(1b) : Outport  Port=43
  Select_20(1b) : Outport  Port=44
  Select_19(1b) : Outport  Port=45
  Select_18(1b) : Outport  Port=46
  Select_17(1b) : Outport  Port=47
  Select_16(1b) : Outport  Port=48
  Select_15(1b) : Outport  Port=49
  Select_14(1b) : Outport  Port=50
  Select_13(1b) : Outport  Port=51
  Select_12(1b) : Outport  Port=52
  Select_11(1b) : Outport  Port=53
  Select_10(1b) : Outport  Port=54
  Select_09(1b) : Outport  Port=55
  Select_08(1b) : Outport  Port=56
  Select_07(1b) : Outport  Port=57
  Select_06(1b) : Outport  Port=58
  Select_05(1b) : Outport  Port=59
  Select_04(1b) : Outport  Port=60
  Select_03(1b) : Outport  Port=61
  Select_02(1b) : Outport  Port=62
  Select_01(1b) : Outport  Port=63
  Select_00(1b) : Outport  Port=64
  wires:
  Logical Operator16/1 -> Select_48(1b)/1
  Logical Operator15/1 -> Select_49(1b)/1
  Logical Operator14/1 -> Select_50(1b)/1
  Logical Operator13/1 -> Select_51(1b)/1
  Logical Operator12/1 -> Select_52(1b)/1
  Logical Operator11/1 -> Select_53(1b)/1
  Logical Operator10/1 -> Select_54(1b)/1
  Logical Operator9/1 -> Select_55(1b)/1
  Logical Operator8/1 -> Select_56(1b)/1
  Logical Operator7/1 -> Select_57(1b)/1
  Logical Operator6/1 -> Select_58(1b)/1
  Logical Operator4/1 -> Select_59(1b)/1
  Logical Operator1/1 -> Select_62(1b)/1
  Logical Operator2/1 -> Select_61(1b)/1
  Logical Operator3/1 -> Select_60(1b)/1
  Logical Operator/1 -> Select_63(1b)/1
  Logical Operator34/1 -> Select_32(1b)/1
  Logical Operator33/1 -> Select_33(1b)/1
  Logical Operator32/1 -> Select_34(1b)/1
  Logical Operator31/1 -> Select_35(1b)/1
  Logical Operator30/1 -> Select_36(1b)/1
  Logical Operator29/1 -> Select_37(1b)/1
  Logical Operator28/1 -> Select_38(1b)/1
  Logical Operator27/1 -> Select_39(1b)/1
  Logical Operator26/1 -> Select_40(1b)/1
  Logical Operator25/1 -> Select_41(1b)/1
  Logical Operator24/1 -> Select_42(1b)/1
  Logical Operator21/1 -> Select_43(1b)/1
  Logical Operator18/1 -> Select_46(1b)/1
  Logical Operator19/1 -> Select_45(1b)/1
  Logical Operator20/1 -> Select_44(1b)/1
  Logical Operator17/1 -> Select_47(1b)/1
  xyz1/3 -> Lo`gical Operator2/1, Logical Operator/2, Logical Operator1/2, Logical Operator2/2, Logical Operator3/2, Logical Operator4/2, Logical Operator6/2, Logical Operator7/2, Logical Operator9/2, Logical Operator10/2, Logical Operator11/2, Logical Operator12/2, Logical Operator13/2, Logical Operator14/2, Logical Operator15/2, Logical Operator16/2, Logical Operator17/2, Logical Operator18/2, Logical Operator19/2, Logical Operator20/2, Logical Operator21/2, Logical Operator24/2, Logical Operator25/2, Logical Operator26/2, Logical Operator27/2, Logical Operator28/2, Logical Operator29/2, Logical Operator30/2, Logical Operator31/2, Logical Operator32/2, Logical Operator33/2, Logical Operator34/2, Logical Operator8/2  "<signal3>"
  Logical Operator43/1 -> Select_16(1b)/1
  Logical Operator42/1 -> Select_17(1b)/1
  Logical Operator41/1 -> Select_18(1b)/1
  Logical Operator40/1 -> Select_19(1b)/1
  Logical Operator39/1 -> Select_20(1b)/1
  Logical Operator38/1 -> Select_21(1b)/1
  Logical Operator37/1 -> Select_22(1b)/1
  Logical Operator69/1 -> Select_23(1b)/1
  Logical Operator68/1 -> Select_24(1b)/1
  Logical Operator67/1 -> Select_25(1b)/1
  Logical Operator66/1 -> Select_26(1b)/1
  Logical Operator64/1 -> Select_27(1b)/1
  Logical Operator36/1 -> Select_30(1b)/1
  Logical Operator47/1 -> Select_29(1b)/1
  Logical Operator58/1 -> Select_28(1b)/1
  Logical Operator35/1 -> Select_31(1b)/1
  Logical Operator22/1 -> Logical Operator2/6, Logical Operator3/6, Logical Operator7/6, Logical Operator8/6, Logical Operator11/6, Logical Operator12/6, Logical Operator15/6, Logical Operator63/6, Logical Operator62/6, Logical Operator59/6, Logical Operator57/6, Logical Operator54/6, Logical Operator53/6, Logical Operator48/6, Logical Operator46/6, Logical Operator43/6, Logical Operator42/6, Logical Operator39/6, Logical Operator38/6, Logical Operator68/6, Logical Operator67/6, Logical Operator58/6, Logical Operator47/6, Logical Operator34/6, Logical Operator33/6, Logical Operator30/6, Logical Operator29/6, Logical Operator26/6, Logical Operator25/6, Logical Operator20/6, Logical Operator19/6, Logical Operator16/6
  xyz1/8 -> Logical Operator/7, Logical Operator62/7, Logical Operator60/7, Logical Operator57/7, Logical Operator55/7, Logical Operator53/7, Logical Operator49/7, Logical Operator46/7, Logical Operator44/7, Logical Operator42/7, Logical Operator40/7, Logical Operator38/7, Logical Operator69/7, Logical Operator67/7, Logical Operator64/7, Logical Operator47/7, Logical Operator35/7, Logical Operator33/7, Logical Operator31/7, Logical Operator29/7, Logical Operator27/7, Logical Operator25/7, Logical Operator21/7, Logical Operator19/7, Logical Operator17/7, Logical Operator15/7, Logical Operator13/7, Logical Operator11/7, Logical Operator9/7, Logical Operator7/7, Logical Operator4/7, Logical Operator2/7, Logical Operator23/1  "<signal8>"
  Logical Operator23/1 -> Logical Operator43/7, Logical Operator56/7, Logical Operator63/7, Logical Operator61/7, Logical Operator59/7, Logical Operator54/7, Logical Operator52/7, Logical Operator48/7, Logical Operator45/7, Logical Operator41/7, Logical Operator39/7, Logical Operator37/7, Logical Operator68/7, Logical Operator66/7, Logical Operator58/7, Logical Operator36/7, Logical Operator34/7, Logical Operator32/7, Logical Operator30/7, Logical Operator28/7, Logical Operator26/7, Logical Operator24/7, Logical Operator20/7, Logical Operator18/7, Logical Operator16/7, Logical Operator14/7, Logical Operator12/7, Logical Operator10/7, Logical Operator8/7, Logical Operator6/7, Logical Operator3/7, Logical Operator1/7
  Logical Operator5/1 -> Logical Operator43/5, Logical Operator63/5, Logical Operator62/5, Logical Operator61/5, Logical Operator60/5, Logical Operator54/5, Logical Operator53/5, Logical Operator52/5, Logical Operator49/5, Logical Operator42/5, Logical Operator41/5, Logical Operator40/5, Logical Operator68/5, Logical Operator67/5, Logical Operator66/5, Logical Operator64/5, Logical Operator34/5, Logical Operator33/5, Logical Operator32/5, Logical Operator31/5, Logical Operator26/5, Logical Operator25/5, Logical Operator24/5, Logical Operator21/5, Logical Operator16/5, Logical Operator15/5, Logical Operator14/5, Logical Operator13/5, Logical Operator8/5, Logical Operator7/5, Logical Operator6/5, Logical Operator4/5
  xyz1/5 -> Lo`gical Operator4/1, Logical Operator/4, Logical Operator1/4, Logical Operator2/4, Logical Operator3/4, Logical Operator4/4, Logical Operator7/4, Logical Operator8/4, Logical Operator17/4, Logical Operator18/4, Logical Operator19/4, Logical Operator20/4, Logical Operator21/4, Logical Operator24/4, Logical Operator25/4, Logical Operator26/4, Logical Operator35/4, Logical Operator36/4, Logical Operator47/4, Logical Operator58/4, Logical Operator64/4, Logical Operator66/4, Logical Operator67/4, Logical Operator68/4, Logical Operator44/4, Logical Operator45/4, Logical Operator46/4, Logical Operator48/4, Logical Operator49/4, Logical Operator52/4, Logical Operator54/4, Logical Operator53/4, Logical Operator6/4  "<signal5>"
  xyz1/7 -> Logical Operator22/1, Logical Operator1/6, Logical Operator4/6, Logical Operator6/6, Logical Operator9/6, Logical Operator10/6, Logical Operator13/6, Logical Operator14/6, Logical Operator17/6, Logical Operator18/6, Logical Operator21/6, Logical Operator24/6, Logical Operator27/6, Logical Operator28/6, Logical Operator31/6, Logical Operator32/6, Logical Operator35/6, Logical Operator36/6, Logical Operator64/6, Logical Operator66/6, Logical Operator69/6, Logical Operator37/6, Logical Operator40/6, Logical Operator41/6, Logical Operator44/6, Logical Operator45/6, Logical Operator49/6, Logical Operator52/6, Logical Operator55/6, Logical Operator56/6, Logical Operator61/6, Logical Operator60/6, Logical Operator/6  "<signal7>"
  xyz1/6 -> Logical Operator/5, Logical Operator1/5, Logical Operator3/5, Logical Operator9/5, Logical Operator10/5, Logical Operator11/5, Logical Operator12/5, Logical Operator17/5, Logical Operator18/5, Logical Operator20/5, Logical Operator27/5, Logical Operator28/5, Logical Operator29/5, Logical Operator30/5, Logical Operator35/5, Logical Operator36/5, Logical Operator69/5, Logical Operator37/5, Logical Operator38/5, Logical Operator39/5, Logical Operator44/5, Logical Operator45/5, Logical Operator48/5, Logical Operator55/5, Logical Operator56/5, Logical Operator59/5, Logical Operator57/5, Logical Operator46/5, Logical Operator58/5, Logical Operator47/5, Logical Operator19/5, Logical Operator2/5, Logical Operator5/1  "<signal6>"
  Lo`gical Operator4/1 -> Logical Operator9/4, Logical Operator10/4, Logical Operator11/4, Logical Operator12/4, Logical Operator13/4, Logical Operator14/4, Logical Operator15/4, Logical Operator27/4, Logical Operator28/4, Logical Operator29/4, Logical Operator30/4, Logical Operator31/4, Logical Operator32/4, Logical Operator33/4, Logical Operator69/4, Logical Operator37/4, Logical Operator38/4, Logical Operator39/4, Logical Operator40/4, Logical Operator41/4, Logical Operator42/4, Logical Operator43/4, Logical Operator56/4, Logical Operator57/4, Logical Operator60/4, Logical Operator61/4, Logical Operator62/4, Logical Operator63/4, Logical Operator59/4, Logical Operator55/4, Logical Operator34/4, Logical Operator16/4
  Logical Operator63/1 -> Select_00(1b)/1
  Logical Operator62/1 -> Select_01(1b)/1
  Logical Operator61/1 -> Select_02(1b)/1
  Logical Operator60/1 -> Select_03(1b)/1
  Logical Operator59/1 -> Select_04(1b)/1
  Logical Operator57/1 -> Select_05(1b)/1
  Logical Operator56/1 -> Select_06(1b)/1
  Logical Operator55/1 -> Select_07(1b)/1
  Logical Operator54/1 -> Select_08(1b)/1
  Logical Operator53/1 -> Select_09(1b)/1
  Logical Operator52/1 -> Select_10(1b)/1
  Logical Operator49/1 -> Select_11(1b)/1
  Logical Operator45/1 -> Select_14(1b)/1
  Logical Operator46/1 -> Select_13(1b)/1
  Logical Operator48/1 -> Select_12(1b)/1
  Logical Operator44/1 -> Select_15(1b)/1
  Lo`gical Operator1/1 -> Logical Operator63/3, Logical Operator62/3, Logical Operator61/3, Logical Operator60/3, Logical Operator59/3, Logical Operator57/3, Logical Operator56/3, Logical Operator55/3, Logical Operator54/3, Logical Operator53/3, Logical Operator52/3, Logical Operator49/3, Logical Operator48/3, Logical Operator46/3, Logical Operator45/3, Logical Operator44/3, Logical Operator34/3, Logical Operator33/3, Logical Operator32/3, Logical Operator31/3, Logical Operator30/3, Logical Operator29/3, Logical Operator28/3, Logical Operator27/3, Logical Operator26/3, Logical Operator25/3, Logical Operator24/3, Logical Operator21/3, Logical Operator20/3, Logical Operator19/3, Logical Operator18/3, Logical Operator17/3
  xyz1/4 -> Logical Operator8/3, Logical Operator47/3, Logical Operator43/3, Logical Operator42/3, Logical Operator41/3, Logical Operator40/3, Logical Operator39/3, Logical Operator38/3, Logical Operator37/3, Logical Operator69/3, Logical Operator68/3, Logical Operator67/3, Logical Operator66/3, Logical Operator64/3, Logical Operator58/3, Logical Operator36/3, Logical Operator35/3, Logical Operator16/3, Logical Operator15/3, Logical Operator14/3, Logical Operator13/3, Logical Operator12/3, Logical Operator11/3, Logical Operator10/3, Logical Operator9/3, Logical Operator7/3, Logical Operator6/3, Logical Operator4/3, Logical Operator3/3, Logical Operator2/3, Logical Operator1/3, Logical Operator/3, Lo`gical Operator1/1  "<signal4>"
  Enable(1b)/1 -> Logical Operator/1, Logical Operator1/1, Logical Operator2/1, Logical Operator3/1, Logical Operator4/1, Logical Operator6/1, Logical Operator7/1, Logical Operator8/1, Logical Operator9/1, Logical Operator10/1, Logical Operator11/1, Logical Operator12/1, Logical Operator13/1, Logical Operator14/1, Logical Operator15/1, Logical Operator16/1, Logical Operator17/1, Logical Operator18/1, Logical Operator19/1, Logical Operator20/1, Logical Operator21/1, Logical Operator24/1, Logical Operator25/1, Logical Operator26/1, Logical Operator27/1, Logical Operator28/1, Logical Operator29/1, Logical Operator30/1, Logical Operator31/1, Logical Operator32/1, Logical Operator33/1, Logical Operator35/1, Logical Operator36/1, Logical Operator47/1, Logical Operator58/1, Logical Operator64/1, Logical Operator66/1, Logical Operator67/1, Logical Operator68/1, Logical Operator69/1, Logical Operator37/1, Logical Operator38/1, Logical Operator39/1, Logical Operator40/1, Logical Operator41/1, Logical Operator42/1, Logical Operator43/1, Logical Operator44/1, Logical Operator45/1, Logical Operator46/1, Logical Operator48/1, Logical Operator49/1, Logical Operator52/1, Logical Operator53/1, Logical Operator54/1, Logical Operator55/1, Logical Operator56/1, Logical Operator57/1, Logical Operator59/1, Logical Operator60/1, Logical Operator61/1, Logical Operator62/1, Logical Operator63/1, Logical Operator34/1
  Lo`gical Operator2/1 -> Logical Operator35/2, Logical Operator36/2, Logical Operator58/2, Logical Operator64/2, Logical Operator66/2, Logical Operator67/2, Logical Operator68/2, Logical Operator69/2, Logical Operator37/2, Logical Operator38/2, Logical Operator39/2, Logical Operator40/2, Logical Operator41/2, Logical Operator42/2, Logical Operator43/2, Logical Operator44/2, Logical Operator45/2, Logical Operator46/2, Logical Operator48/2, Logical Operator49/2, Logical Operator52/2, Logical Operator53/2, Logical Operator54/2, Logical Operator55/2, Logical Operator56/2, Logical Operator57/2, Logical Operator59/2, Logical Operator60/2, Logical Operator61/2, Logical Operator62/2, Logical Operator63/2, Logical Operator47/2
  Addr(1B)/1 -> xyz1/1
  xyz1/1 -> Terminator/1  "<signal1>"
  xyz1/2 -> Terminator1/1  "<signal2>"
